simulink model "TosAeb"  (R2021a)
settings: Solver=FixedStepDiscrete  StartTime=0.0  StopTime=inf  FixedStep=0.01  RelTol=1e-3
undefined symbols (not defined in the file or in its folder): ACCModSts, AccSts1, AEB_ObjectMovingOrientation, AEB_ObjectMovingState, CN_BTCActive, CN_CurrentGearR, CN_CurrentGearR_DHT, CN_PTCActive, CN_VDCActive, CN_VSC_EspOff, CN_VSC_FCW_AEB_Error, CN_VSC_FCW_AEB_NoDispay, CN_VSC_FCW_AEB_PerfmDgdtn, CN_VSC_FCW_AEB_TempUnAvlbl, CN_VSC_VehStandstill, CNaccelAvoidMax, CNaccelAvoidMin, CNbrakePedalPress, CNcomfortStrLeftAccelMax, CNcomfortStrLeftAccelMin, CNcomfortStrRightAccelMax, CNcomfortStrRightAccelMin, CNDrvDoorOpen, CNDrvModeEco, CNDrvModeNorm, CNDrvModeOther, CNDrvModeSport, CNDrvSBRreminder, CNNull, CNobjInvalidID, CNPassDoorOpen, CNPassSBRreminder, CNPEBSAccelNegInf, CNPEBSAccelNull, CNPEBSTimeInf, CNPEBSTimeNull, CNSTOPMAX, CNSTOPNULL, CNsubjWidth, CNSwtOFF, CNSwtON, CNTTCMAX, CNTTCNULL, CNVehConfigAEBCycNone, CNVehConfigAEBNone, CNVehConfigAEBPedNone, CNVehConfigFCWNone, CNVehConfigJANone, CollisionSeverity, FALSE, FCWSnvty, JACycObjCalcInfoDefaultParams, JAPedObjCalcInfoDefaultParams, JAVehObjCalcInfoDefaultParams, latCycObjCalcInfoDefaultParams, latPedObjCalcInfoDefaultParams, longObjCalcInfoDefaultParams, ModSts, movingStatus, ObjectType ... (+1112)
libraries: simulink x3031, AEBSFunctionLib x50, Ta_Library x33, AEBSStateMachineLib x23, Mylibrary x12, simulink_extras x7, ObjSelect_lib x3, dspstat3 x2
(part 9 of 102)

== system TosAeb/FcwAebJa_Step_sys/ALG_AEBSystem/ALG_AEBController/AEB_StateMachineCalcApp/JA_AEBStateMachine ==
  note: Add New
  InputSignal : Inport
  JA_PTW_AEBfullStateMachine : Subsystem
  JA_PTW_PrefillStateMachine : Subsystem
  JA_cycAEBfullStateMachine : Subsystem
  JA_cycPrefillStateMachine : Subsystem
  JA_pedAEBfullStateMachine : Subsystem
  JA_pedPrefillStateMachine : Subsystem
  JA_vehAEBStateMachine : Subsystem
  JA_vehPrefillStateMachine : Subsystem
  Bus Element Out : Outport  PortName=JA_AEBRawModSts  Element=JA_vehPrefillRawModSts
  Bus Element Out1 : Outport  PortName=JA_AEBRawModSts  Element=JA_vehAEBRawModSts
  Bus Element Out2 : Outport  PortName=JA_AEBRawModSts  Element=JA_pedPrefillRawModSts
  Bus Element Out3 : Outport  PortName=JA_AEBRawModSts  Element=JA_pedAEBRawModSts
  Bus Element Out8 : Outport  PortName=JA_AEBRawModSts  Element=JA_cycPrefillRawModSts
  Bus Element Out9 : Outport  PortName=JA_AEBRawModSts  Element=JA_cycAEBRawModSts
  Bus Element Out12 : Outport  PortName=JA_AEBRawModSts  Element=JA_PTW_PrefillRawModSts
  Bus Element Out13 : Outport  PortName=JA_AEBRawModSts  Element=JA_PTW_AEBRawModSts
  Bus Element Out4 : Outport  Port=2  PortName=JA_AEBModSts  Element=JA_vehPrefillModSts
  Bus Element Out5 : Outport  Port=2  PortName=JA_AEBModSts  Element=JA_vehAEBModSts
  Bus Element Out6 : Outport  Port=2  PortName=JA_AEBModSts  Element=JA_pedPrefillModSts
  Bus Element Out7 : Outport  Port=2  PortName=JA_AEBModSts  Element=JA_pedAEBModSts
  Bus Element Out10 : Outport  Port=2  PortName=JA_AEBModSts  Element=JA_cycPrefillModSts
  Bus Element Out11 : Outport  Port=2  PortName=JA_AEBModSts  Element=JA_cycAEBModSts
  Bus Element Out14 : Outport  Port=2  PortName=JA_AEBModSts  Element=JA_PTW_PrefillModSts
  Bus Element Out15 : Outport  Port=2  PortName=JA_AEBModSts  Element=JA_PTW_AEBModSts
  wires:
  InputSignal/1 -> JA_vehAEBStateMachine/1(inputSignal), JA_pedPrefillStateMachine/1(inputSignal), JA_cycPrefillStateMachine/1(inputSignal), JA_cycAEBfullStateMachine/1(inputSignal), JA_PTW_AEBfullStateMachine/1(inputSignal), JA_PTW_PrefillStateMachine/1(inputSignal), JA_pedAEBfullStateMachine/1(inputSignal), JA_vehPrefillStateMachine/1(inputSignal)
  JA_vehPrefillStateMachine/2(JA_vehPrefillModSts) -> Bus Element Out4/1  "JA_vehPrefillModSts"
  JA_vehAEBStateMachine/2(JA_vehAEBModSts) -> Bus Element Out5/1  "JA_vehAEBModSts"
  JA_pedAEBfullStateMachine/2(JA_pedAEBModSts) -> Bus Element Out7/1  "JA_pedAEBModSts"
  JA_pedPrefillStateMachine/2(JA_pedPrefillModSts) -> Bus Element Out6/1  "JA_pedPrefillModSts"
  JA_vehPrefillStateMachine/1(JA_vehPrefillRawModSts) -> Bus Element Out/1
  JA_vehAEBStateMachine/1(JA_vehAEBRawModSts) -> Bus Element Out1/1
  JA_pedPrefillStateMachine/1(JA_pedPrefillRawModSts) -> Bus Element Out2/1
  JA_pedAEBfullStateMachine/1(JA_pedAEBRawModSts) -> Bus Element Out3/1
  JA_cycAEBfullStateMachine/1(JA_cycAEBRawModSts) -> Bus Element Out9/1
  JA_cycPrefillStateMachine/1(JA_cycPrefillRawModSts) -> Bus Element Out8/1
  JA_cycPrefillStateMachine/2(JA_cycPrefillModSts) -> Bus Element Out10/1
  JA_cycAEBfullStateMachine/2(JA_cycAEBModSts) -> Bus Element Out11/1
  JA_PTW_AEBfullStateMachine/2(JA_PTW_AEBModSts) -> Bus Element Out15/1
  JA_PTW_PrefillStateMachine/2(JA_PTW_PrefillModSts) -> Bus Element Out14/1
  JA_PTW_PrefillStateMachine/1(JA_PTW_PrefillRawModSts) -> Bus Element Out12/1
  JA_PTW_AEBfullStateMachine/1(JA_PTW_AEBRawModSts) -> Bus Element Out13/1

== system TosAeb/FcwAebJa_Step_sys/ALG_AEBSystem/ALG_AEBController/AEB_StateMachineCalcApp/JaFctStsArb ==
  JA_AEBModSts : Inport
  BrkArb : Subsystem
  BrkReadyArb : Subsystem
  Bus Selector : BusSelector  OutputSignals=JA_vehAEBModSts,JA_pedAEBModSts,JA_cycAEBModSts,JA_PTW_AEBModSts
  Bus Selector2 : BusSelector  OutputSignals=JA_vehPrefillModSts,JA_pedPrefillModSts,JA_cycPrefillModSts,JA_PTW_PrefillModSts
  Bus Element Out : Outport  PortName=JA_AEBModStsArb  Element=JA_vehAEBModStsArb
  Bus Element Out1 : Outport  PortName=JA_AEBModStsArb  Element=JA_PTW_AEBModStsArb
  Bus Element Out2 : Outport  PortName=JA_AEBModStsArb  Element=JA_cycAEBModStsArb
  Bus Element Out3 : Outport  PortName=JA_AEBModStsArb  Element=JA_pedAEBModStsArb
  Bus Element Out4 : Outport  PortName=JA_AEBModStsArb  Element=JA_vehPrefillModStsArb
  Bus Element Out5 : Outport  PortName=JA_AEBModStsArb  Element=JA_PTW_PrefillModStsArb
  Bus Element Out6 : Outport  PortName=JA_AEBModStsArb  Element=JA_cycPrefillModStsArb
  Bus Element Out7 : Outport  PortName=JA_AEBModStsArb  Element=JA_pedPrefillModStsArb
  Bus Element Out8 : Outport  Port=2  PortName=vehFctStsArbSuppAborCond  Element=brkSuppAborCondArb
  Bus Element Out9 : Outport  Port=2  PortName=vehFctStsArbSuppAborCond  Element=brkReadySuppAborCondArb
  wires:
  BrkArb/5(brkSuppAborCondArb) -> Bus Element Out8/1  "brkSuppAborCondArb"
  BrkArb/6(brkActvCond) -> BrkReadyArb/1(brkActvCond)
  JA_AEBModSts/1 -> Bus Selector/1, Bus Selector2/1
  Bus Selector/4 -> BrkArb/4(JA_PTW_AEBModSts)  "<JA_PTW_AEBModSts>"
  Bus Selector/3 -> BrkArb/3(JA_cycAEBModSts)  "<JA_cycAEBModSts>"
  Bus Selector/2 -> BrkArb/2(JA_pedAEBModSts)  "<JA_pedAEBModSts>"
  Bus Selector/1 -> BrkArb/1(JA_vehAEBModSts)  "<JA_vehAEBModSts>"
  Bus Selector2/1 -> BrkReadyArb/2(JA_vehPrefillModSts)  "<JA_vehPrefillModSts>"
  Bus Selector2/2 -> BrkReadyArb/3(JA_pedPrefillModSts)  "<JA_pedPrefillModSts>"
  Bus Selector2/3 -> BrkReadyArb/4(JA_cycPrefillModSts)  "<JA_cycPrefillModSts>"
  Bus Selector2/4 -> BrkReadyArb/5(JA_PTW_PrefillModSts)  "<JA_PTW_PrefillModSts>"
  BrkReadyArb/1(brkReadySuppAborCondArb) -> Bus Element Out9/1  "brkReadySuppAborCondArb"
  BrkArb/4(JA_pedAEBModStsArb) -> Bus Element Out3/1  "JA_pedAEBModStsArb"
  BrkArb/1(JA_vehAEBModStsArb) -> Bus Element Out/1  "JA_vehAEBModStsArb"
  BrkArb/2(JA_PTW_AEBModStsArb) -> Bus Element Out1/1  "JA_PTW_AEBModStsArb"
  BrkArb/3(JA_cycAEBModStsArb) -> Bus Element Out2/1  "JA_cycAEBModStsArb"
  BrkReadyArb/2(JA_vehPrefillModStsArb) -> Bus Element Out4/1  "JA_vehPrefillModStsArb"
  BrkReadyArb/3(JA_PTW_PrefillModStsArb) -> Bus Element Out5/1  "JA_PTW_PrefillModStsArb"
  BrkReadyArb/4(JA_cycPrefillModStsArb) -> Bus Element Out6/1  "JA_cycPrefillModStsArb"
  BrkReadyArb/5(JA_pedPrefillModStsArb) -> Bus Element Out7/1  "JA_pedPrefillModStsArb"

== system TosAeb/FcwAebJa_Step_sys/ALG_AEBSystem/ALG_AEBController/AEB_StateMachineCalcApp/LatAEBStateMachine ==
  InputSignal : Inport
  cycAEBfullStateMachine : Subsystem
  cycAEBpartStateMachine : Subsystem
  cycPrefillStateMachine : Subsystem
  pedABAStateMachine : Subsystem
  pedAEBfullStateMachine : Subsystem
  pedPreWarningStateMachine : Subsystem
  pedPrefillStateMachine : Subsystem
  Bus Element Out8 : Outport  PortName=latAEBRawModSts  Element=pedPrefillRawModSts
  Bus Element Out7 : Outport  PortName=latAEBRawModSts  Element=pedPreWarningRawModSts
  Bus Element Out6 : Outport  PortName=latAEBRawModSts  Element=pedABARawModSts
  Bus Element Out5 : Outport  PortName=latAEBRawModSts  Element=pedAEBfullRawModSts
  Bus Element Out4 : Outport  PortName=latAEBRawModSts  Element=cycPrefillRawModSts
  Bus Element Out3 : Outport  PortName=latAEBRawModSts  Element=cycAEBpartRawModSts
  Bus Element Out2 : Outport  PortName=latAEBRawModSts  Element=cycAEBfullRawModSts
  Bus Element Out : Outport  Port=2  PortName=latAEBModSts  Element=pedPrefillModSts
  Out Bus Element : Outport  Port=2  PortName=latAEBModSts  Element=pedPreWarningModSts
  Out Bus Element1 : Outport  Port=2  PortName=latAEBModSts  Element=pedABAModSts
  Out Bus Element2 : Outport  Port=2  PortName=latAEBModSts  Element=cycPrefillModSts
  Out Bus Element3 : Outport  Port=2  PortName=latAEBModSts  Element=pedAEBfullModSts
  Out Bus Element4 : Outport  Port=2  PortName=latAEBModSts  Element=cycAEBpartModSts
  Out Bus Element5 : Outport  Port=2  PortName=latAEBModSts  Element=cycAEBfullModSts
  Bus Element Out1 : Outport  Port=3  PortName=latActiveStateTime  Element=pedPrefillWorkTime
  Out Bus Element6 : Outport  Port=3  PortName=latActiveStateTime  Element=pedPreWarningWorkTime
  Out Bus Element7 : Outport  Port=3  PortName=latActiveStateTime  Element=pedABAWorkTime
  Out Bus Element8 : Outport  Port=3  PortName=latActiveStateTime  Element=pedAEBfullWorkTime
  Out Bus Element9 : Outport  Port=3  PortName=latActiveStateTime  Element=cycPrefillWorkTime
  Out Bus Element10 : Outport  Port=3  PortName=latActiveStateTime  Element=cycAEBpartWorkTime
  Out Bus Element11 : Outport  Port=3  PortName=latActiveStateTime  Element=cycAEBfullWorkTime
  wires:
  InputSignal/1 -> cycAEBfullStateMachine/1(inputSignal), cycAEBpartStateMachine/1(inputSignal), cycPrefillStateMachine/1(inputSignal), pedAEBfullStateMachine/1(inputSignal), pedABAStateMachine/1(inputSignal), pedPreWarningStateMachine/1(inputSignal), pedPrefillStateMachine/1(inputSignal)
  pedPrefillStateMachine/2(pedPrefillModSts) -> Bus Element Out/1  "pedPrefillModSts"
  pedPreWarningStateMachine/2(pedPreWarningModSts) -> Out Bus Element/1  "pedPreWarningModSts"
  pedABAStateMachine/2(pedABAModSts) -> Out Bus Element1/1  "pedABAModSts"
  cycPrefillStateMachine/2(cycPrefillModSts) -> Out Bus Element2/1  "cycPrefillModSts"
  pedAEBfullStateMachine/2(pedAEBfullModSts) -> Out Bus Element3/1  "pedAEBfullModSts"
  cycAEBpartStateMachine/2(cycAEBpartModSts) -> Out Bus Element4/1  "cycAEBpartModSts"
  cycAEBfullStateMachine/2(cycAEBfullModSts) -> Out Bus Element5/1  "cycAEBfullModSts"
  pedPrefillStateMachine/3(pedPrefillFuncActiveTime) -> Bus Element Out1/1  "pedPrefillFuncActiveTime"
  pedPreWarningStateMachine/3(pedPreWarningFuncActiveTime) -> Out Bus Element6/1
  pedABAStateMachine/3(pedABAFuncActiveTime) -> Out Bus Element7/1
  cycAEBpartStateMachine/3(cycAEBpartFuncActiveTime) -> Out Bus Element10/1
  cycAEBfullStateMachine/3(cycAEBfullFuncActiveTime) -> Out Bus Element11/1
  cycPrefillStateMachine/3(cycPrefillFuncActiveTime) -> Out Bus Element9/1
  pedAEBfullStateMachine/3(pedAEBfullFuncActiveTime) -> Out Bus Element8/1
  cycAEBfullStateMachine/1(cycAEBfullRawModSts) -> Bus Element Out2/1
  cycAEBpartStateMachine/1(cycAEBpartRawModSts) -> Bus Element Out3/1
  cycPrefillStateMachine/1(cycPrefillRawModSts) -> Bus Element Out4/1
  pedAEBfullStateMachine/1(pedAEBfullRawModSts) -> Bus Element Out5/1
  pedABAStateMachine/1(pedABARawModSts) -> Bus Element Out6/1
  pedPreWarningStateMachine/1(pedPreWarningRawModSts) -> Bus Element Out7/1
  pedPrefillStateMachine/1(pedPrefillRawModSts) -> Bus Element Out8/1

== system TosAeb/FcwAebJa_Step_sys/ALG_AEBSystem/ALG_AEBController/AEB_StateMachineCalcApp/LatFctStsArb ==
  latAEBModSts : Inport
  BrkArb : Subsystem
  BrkReadyArb : Subsystem
  Bus Creator1 : BusCreator  Inputs=7
  Bus Creator2 : BusCreator  Inputs=3
  WarningArb : Subsystem
  latAEBModStsArb : Outport
  latFctStsArbSuppAborCond : Outport  Port=2
  wires:
  [brkActvCond] -> WarningArb/2(brkActvCond)
  Bus Creator1/1 -> latAEBModStsArb/1  "latAEBModStsArb"
  Bus Creator2/1 -> latFctStsArbSuppAborCond/1  "latFctStsArbSuppAborCond"
  BrkArb/5(brkSuppAborCondArb) -> Bus Creator2/3  "brkSuppAborCondArb"
  [brkReadySuppAborCond] -> Bus Creator2/1  "brkReadySuppAborCond"
  WarningArb/2(warningSuppAborCond) -> [warningSuppAborCond]
  [warningSuppAborCond] -> Bus Creator2/2  "warningSuppAborCond"
  BrkReadyArb/4(brkReadySuppAborCond) -> [brkReadySuppAborCond]
  BrkArb/1(brkActvCond) -> BrkReadyArb/2(brkActvCond), [brkActvCond]
  latAEBModSts/1 -> WarningArb/1(vehAEBModSts), BrkArb/1(vehAEBModSts), BrkReadyArb/1(latAEBModSts)
  WarningArb/1(pedPreWarningModStsArb) -> Bus Creator1/1  "pedPreWarningModStsArb"
  BrkReadyArb/1(pedPrefillModStsArb) -> Bus Creator1/2  "pedPrefillModStsArb"
  BrkReadyArb/2(cycPrefillModStsArb) -> Bus Creator1/3  "cycPrefillModStsArb"
  BrkReadyArb/3(pedABAModStsArb) -> Bus Creator1/4  "pedABAModStsArb"
  BrkArb/4(pedAEBfullModStsArb) -> Bus Creator1/7  "pedAEBfullModStsArb"
  BrkArb/3(cycAEBfullModStsArb) -> Bus Creator1/6  "cycAEBfullModStsArb"
  BrkArb/2(cycAEBpartModStsArb) -> Bus Creator1/5  "cycAEBpartModStsArb"

== system TosAeb/FcwAebJa_Step_sys/ALG_AEBSystem/ALG_AEBController/AEB_StateMachineCalcApp/VehAEBStateMachine ==
  InputSignal : Inport
  ABAStateMachine : Subsystem
  AEBfullStateMachine : Subsystem
  AEBpartStateMachine : Subsystem
  ActuteWarningStateMachine : Subsystem
  EBAStateMachine : Subsystem
  LatentWarningStateMachine : Subsystem
  PreWarningStateMachine : Subsystem
  PrefillStateMachine : Subsystem
  Bus Element Out : Outport  PortName=vehRawModSts  Element=vehPrefillRawModSts
  Bus Element Out1 : Outport  PortName=vehRawModSts  Element=vehPreWarningRawModSts
  Bus Element Out2 : Outport  PortName=vehRawModSts  Element=vehAcuteWarningRawModSts
  Bus Element Out3 : Outport  PortName=vehRawModSts  Element=vehAEBpartRawModSts
  Bus Element Out4 : Outport  PortName=vehRawModSts  Element=vehAEBfullRawModSts
  Bus Element Out5 : Outport  PortName=vehRawModSts  Element=vehEBARawModSts
  Bus Element Out6 : Outport  PortName=vehRawModSts  Element=vehABARawModSts
  Bus Element Out7 : Outport  PortName=vehRawModSts  Element=vehLatentWarningRawModSts
  Bus Element Out8 : Outport  Port=2  PortName=vehAEBModSts  Element=vehPrefillModSts
  Bus Element Out9 : Outport  Port=2  PortName=vehAEBModSts  Element=vehPreWarningModSts
  Bus Element Out10 : Outport  Port=2  PortName=vehAEBModSts  Element=vehAcuteWarningModSts
  Bus Element Out11 : Outport  Port=2  PortName=vehAEBModSts  Element=vehAEBpartModSts
  Bus Element Out12 : Outport  Port=2  PortName=vehAEBModSts  Element=vehAEBfullModSts
  Bus Element Out13 : Outport  Port=2  PortName=vehAEBModSts  Element=vehEBAModSts
  Bus Element Out14 : Outport  Port=2  PortName=vehAEBModSts  Element=vehABAModSts
  Bus Element Out15 : Outport  Port=2  PortName=vehAEBModSts  Element=vehLatentWarningModSts
  wires:
  InputSignal/1 -> PreWarningStateMachine/1(inputSignal), ActuteWarningStateMachine/1(inputSignal), AEBpartStateMachine/1(inputSignal), AEBfullStateMachine/1(inputSignal), EBAStateMachine/1(inputSignal), LatentWarningStateMachine/1(inputSignal), ABAStateMachine/1(inputSignal), PrefillStateMachine/1(inputSignal)
  LatentWarningStateMachine/2(vehLatentWarningModSts) -> Bus Element Out15/1  "vehLatentWarningModSts"
  PrefillStateMachine/2(vehPrefillModSts) -> Bus Element Out8/1  "vehPrefillModSts"
  PreWarningStateMachine/2(vehPreWarningModSts) -> Bus Element Out9/1  "vehPreWarningModSts"
  ABAStateMachine/2(vehABAModSts) -> Bus Element Out14/1  "vehABAModSts"
  AEBfullStateMachine/2(vehAEBfullModSts) -> Bus Element Out12/1  "vehAEBfullModSts"
  AEBpartStateMachine/2(vehAEBpartModSts) -> Bus Element Out11/1  "vehAEBpartModSts"
  ActuteWarningStateMachine/2(vehAcuteWarningModSts) -> Bus Element Out10/1  "vehAcuteWarningModSts"
  EBAStateMachine/2(vehEBAModSts) -> Bus Element Out13/1  "vehEBAModSts"
  PrefillStateMachine/1(vehPrefillRawModSts) -> Bus Element Out/1
  PreWarningStateMachine/1(vehPreWarningRawModSts) -> Bus Element Out1/1
  ActuteWarningStateMachine/1(vehAcuteWarningRawModSts) -> Bus Element Out2/1
  AEBpartStateMachine/1(vehAEBpartRawModSts) -> Bus Element Out3/1
  AEBfullStateMachine/1(vehAEBfullRawModSts) -> Bus Element Out4/1
  EBAStateMachine/1(vehEBARawModSts) -> Bus Element Out5/1
  ABAStateMachine/1(vehABARawModSts) -> Bus Element Out6/1
  LatentWarningStateMachine/1(vehLatentWarningRawModSts) -> Bus Element Out7/1

== system TosAeb/FcwAebJa_Step_sys/ALG_AEBSystem/ALG_AEBController/AEB_StateMachineCalcApp/VehFCTModStsArb ==
  vehAEBModSts : Inport
  BrkArb : Subsystem
  BrkReadyArb : Subsystem
  Bus Creator1 : BusCreator  Inputs=8
  Bus Creator2 : BusCreator  Inputs=3
  WarningArb : Subsystem
  vehAEBModStsArb : Outport
  vehFctStsArbSuppAborCond : Outport  Port=2
  wires:
  vehAEBModSts/1 -> BrkArb/1(vehAEBModSts), BrkReadyArb/1(VehAEBModSts), WarningArb/1(vehAEBModSts)
  BrkArb/1(brkActvCond) -> [brkActvCond], BrkReadyArb/2(brkActvCond)
  BrkReadyArb/1(brkReadyActvCond) -> WarningArb/2(brkReadyActvCond)
  WarningArb/2(preWarningModStsArb) -> Bus Creator1/2  "preWarningModStsArb"
  BrkArb/4(EBAModStsArb) -> Bus Creator1/8  "EBAModStsArb"
  WarningArb/3(acuteWarningModStsArb) -> Bus Creator1/3  "acuteWarningModStsArb"
  WarningArb/1(latentWarningModStsArb) -> Bus Creator1/1  "latentWarningModStsArb"
  BrkArb/2(AEBpartModStsArb) -> Bus Creator1/6  "AEBpartModStsArb"
  BrkArb/3(AEBfullModStsArb) -> Bus Creator1/7  "AEBfullModStsArb"
  BrkReadyArb/4(brkReadySuppAborCond) -> [brkReadySuppAborCond]
  [warningSuppAborCond] -> Bus Creator2/2  "warningSuppAborCond"
  WarningArb/4(warningSuppAborCond) -> [warningSuppAborCond]
  [brkReadySuppAborCond] -> Bus Creator2/1  "brkReadySuppAborCond"
  BrkArb/5(brkSuppAborCondArb) -> Bus Creator2/3  "brkSuppAborCondArb"
  Bus Creator2/1 -> vehFctStsArbSuppAborCond/1  "FCTStsArbSuppAborCond"
  Bus Creator1/1 -> vehAEBModStsArb/1  "vehAEBModStsArb"
  [brkActvCond] -> WarningArb/3(brkActvCond)
  BrkReadyArb/2(prefillModStsArb) -> Bus Creator1/4  "prefillModStsArb"
  BrkReadyArb/3(ABAModStsArb) -> Bus Creator1/5  "ABAModStsArb"

== system TosAeb/FcwAebJa_Step_sys/ALG_AEBSystem/ALG_AEBController/AEB_VehicleStateCalcApp/CycSuppAborCondtionCalc ==
  InputSignal : Inport
  Bus Creator : BusCreator  Inputs=6  OutDataTypeStr=Bus: BusVSCCycFuncSuppAborCond
      settings: InheritFromInputs=off
  Bus Selector2 : BusSelector  OutputSignals=VSC_AEBFuncSwtSts.AEB_PedResp,VSC_FCW_AEB_FctSts.FCW_AEB_PedFctSts,vehMovingSts.vehUnstableSts,vehMovingSts.vehAssociatedSysStsSuppFlg,vehStateCalcSignalInput.vehConfigInfo.VehicleConfigAEBCyc,vehStateCalcSignalInput.blockTime.blockTimeBrakeReady
  Bus Selector5 : BusSelector  OutputSignals=VSC_AEBFuncSwtSts.AEB_PedResp,VSC_FCW_AEB_FctSts.FCW_AEB_PedFctSts,vehMovingSts.vehUnstableSts,vehMovingSts.vehAssociatedSysStsSuppFlg,vehStateCalcSignalInput.vehConfigInfo.VehicleConfigAEBCyc,vehStateCalcSignalInput.blockTime.blockTimeBrake
  Bus Selector6 : BusSelector  OutputSignals=VSC_AEBFuncSwtSts.AEB_PedResp,VSC_FCW_AEB_FctSts.FCW_AEB_PedFctSts,vehMovingSts.vehUnstableSts,vehMovingSts.vehAssociatedSysStsSuppFlg,vehStateCalcSignalInput.vehConfigInfo.VehicleConfigAEBCyc,vehStateCalcSignalInput.blockTime.blockTimeBrake
  CycAEBfullSuppAborConditonCalc : Subsystem
  CycAEBpartSuppAborConditonCalc : Subsystem
  CycPrefillSuppAborConditonCalc : Subsystem
  VSCCycFuncSuppAborCond : Outport  OutDataTypeStr=Bus: BusVSCCycFuncSuppAborCond
  wires:
  Bus Creator/1 -> VSCCycFuncSuppAborCond/1
  CycPrefillSuppAborConditonCalc/1(VSCCycPrefillSuppCond) -> Bus Creator/1  "VSCCycPrefillSuppCond"
  CycAEBfullSuppAborConditonCalc/2(VSCCycAEBfullAborCond) -> Bus Creator/6  "VSCCycAEBfullAborCond"
  CycPrefillSuppAborConditonCalc/2(VSCCycPrefillAborCond) -> Bus Creator/2  "VSCCycPrefillAborCond"
  CycAEBfullSuppAborConditonCalc/1(VSCCycAEBfullSuppCond) -> Bus Creator/5  "VSCCycAEBfullSuppCond"
  CycAEBpartSuppAborConditonCalc/1(VSCAEBpartSuppCond) -> Bus Creator/3  "VSCAEBpartSuppCond"
  CycAEBpartSuppAborConditonCalc/2(VSCAEBpartAborCond) -> Bus Creator/4  "VSCAEBpartAborCond"
  InputSignal/1 -> Bus Selector6/1, Bus Selector5/1, Bus Selector2/1
  Bus Selector2/1 -> CycPrefillSuppAborConditonCalc/1(AEB_PedResp)  "<AEB_PedResp>"
  Bus Selector2/2 -> CycPrefillSuppAborConditonCalc/2(FCW_AEB_PedFctSts)  "<FCW_AEB_PedFctSts>"
  Bus Selector2/3 -> CycPrefillSuppAborConditonCalc/3(vehUnstableStateFlg)  "<vehUnstableSts>"
  Bus Selector2/4 -> CycPrefillSuppAborConditonCalc/4(vehAssociatedSysStateSuppCond)  "<vehAssociatedSysStsSuppFlg>"
  Bus Selector2/5 -> CycPrefillSuppAborConditonCalc/5("vehConfigAEBCyc ")  "<VehicleConfigAEBCyc>"
  Bus Selector2/6 -> CycPrefillSuppAborConditonCalc/6(blockTimeBrakeReady)  "<blockTimeBrakeReady>"
  Bus Selector5/1 -> CycAEBpartSuppAborConditonCalc/1(VSCAEB_PedResp)  "<AEB_PedResp>"
  Bus Selector5/2 -> CycAEBpartSuppAborConditonCalc/2(FCW_AEB_PedFctSts)  "<FCW_AEB_PedFctSts>"
  Bus Selector5/3 -> CycAEBpartSuppAborConditonCalc/3(vehUnstableStateFlg)  "<vehUnstableSts>"
  Bus Selector5/4 -> CycAEBpartSuppAborConditonCalc/4(vehAssociatedSysStateSuppCond)  "<vehAssociatedSysStsSuppFlg>"
  Bus Selector5/5 -> CycAEBpartSuppAborConditonCalc/5("vehConfigAEBCyc ")  "<VehicleConfigAEBCyc>"
  Bus Selector5/6 -> CycAEBpartSuppAborConditonCalc/6(blockTimeBrake)  "<blockTimeBrake>"
  Bus Selector6/1 -> CycAEBfullSuppAborConditonCalc/1(VSCAEB_PedResp)  "<AEB_PedResp>"
  Bus Selector6/2 -> CycAEBfullSuppAborConditonCalc/2(FCW_AEB_PedFctSts)  "<FCW_AEB_PedFctSts>"
  Bus Selector6/3 -> CycAEBfullSuppAborConditonCalc/3(vehUnstableStateFlg)  "<vehUnstableSts>"
  Bus Selector6/4 -> CycAEBfullSuppAborConditonCalc/4(vehAssociatedSysStateSuppCond)  "<vehAssociatedSysStsSuppFlg>"
  Bus Selector6/5 -> CycAEBfullSuppAborConditonCalc/5("vehConfigAEBCyc ")  "<VehicleConfigAEBCyc>"
  Bus Selector6/6 -> CycAEBfullSuppAborConditonCalc/6(blockTimeBrakeReady)  "<blockTimeBrake>"

== system TosAeb/FcwAebJa_Step_sys/ALG_AEBSystem/ALG_AEBController/AEB_VehicleStateCalcApp/FuncErrorStateJudge ==
  note: 0x0:Trailer off
      | 0x1:Trailer on
  FCW_AEB_ErrorState : Inport
  AEB_Ped_ErrorState : Inport  Port=2
  AEB_JA_ErrorState : Inport  Port=3
  EspFuncOffSts : Inport  Port=4
  TrailerSts : Inport  Port=5
  Bus Creator : BusCreator  Inputs=5
  Bus Selector : BusSelector  OutputSignals=FCW_AEB_ErrorState,EspFuncOffSts,TrailerSts
  Bus Selector1 : BusSelector  OutputSignals=AEB_Ped_ErrorState,EspFuncOffSts,TrailerSts
  Bus Selector2 : BusSelector  OutputSignals=AEB_JA_ErrorState,EspFuncOffSts,TrailerSts
  FCW_AEB_JAFctStsCalc : Stateflow chart  TreatAsAtomicUnit=on
  FCW_AEB_PedFctStsCalc : Stateflow chart  TreatAsAtomicUnit=on
  FCW_AEB_VehFctStsCalc : Stateflow chart  TreatAsAtomicUnit=on
  FCW_AEB_VehFctSts : Outport
  FCW_AEB_PedFctSts : Outport  Port=2
  FCW_AEB_JAFctSts : Outport  Port=3
  wires:
  FCW_AEB_VehFctStsCalc/1(FCW_AEB_VehFctSts) -> FCW_AEB_VehFctSts/1
  FCW_AEB_JAFctStsCalc/1(FCW_AEB_JAFctSts) -> FCW_AEB_JAFctSts/1
  FCW_AEB_PedFctStsCalc/1(FCW_AEB_PedFctSts) -> FCW_AEB_PedFctSts/1
  AEB_Ped_ErrorState/1 -> Bus Creator/2  "AEB_Ped_ErrorState"
  FCW_AEB_ErrorState/1 -> Bus Creator/1  "FCW_AEB_ErrorState"
  AEB_JA_ErrorState/1 -> Bus Creator/3  "AEB_JA_ErrorState"
  EspFuncOffSts/1 -> Bus Creator/4  "EspFuncOffSts"
  TrailerSts/1 -> Bus Creator/5  "TrailerSts"
  Bus Creator/1 -> Bus Selector2/1, Bus Selector1/1, Bus Selector/1
  Bus Selector/1 -> FCW_AEB_VehFctStsCalc/1(FCW_AEB_ErrorState)  "<FCW_AEB_ErrorState>"
  Bus Selector/2 -> FCW_AEB_VehFctStsCalc/2(EspFuncOffSts)  "<EspFuncOffSts>"
  Bus Selector/3 -> FCW_AEB_VehFctStsCalc/3(TrailerSts)  "<TrailerSts>"
  Bus Selector1/1 -> FCW_AEB_PedFctStsCalc/1(FCW_AEB_ErrorState)  "<AEB_Ped_ErrorState>"
  Bus Selector1/2 -> FCW_AEB_PedFctStsCalc/2(EspFuncOffSts)  "<EspFuncOffSts>"
  Bus Selector1/3 -> FCW_AEB_PedFctStsCalc/3(TrailerSts)  "<TrailerSts>"
  Bus Selector2/1 -> FCW_AEB_JAFctStsCalc/1(FCW_AEB_ErrorState)  "<AEB_JA_ErrorState>"
  Bus Selector2/2 -> FCW_AEB_JAFctStsCalc/2(EspFuncOffSts)  "<EspFuncOffSts>"
  Bus Selector2/3 -> FCW_AEB_JAFctStsCalc/3(TrailerSts)  "<TrailerSts>"

== system TosAeb/FcwAebJa_Step_sys/ALG_AEBSystem/ALG_AEBController/AEB_VehicleStateCalcApp/FuncSwtStateJudge ==
  note: 0x00:Normal
      | 0x01:High sensitivity
      | 0x02:Low sensitivity
      | 0x03:Reserved
  AEBVehSwtReq : Inport
  AEBPedSwtReq : Inport  Port=2
  AEBJASwtReq : Inport  Port=3
  FCWSnvtySet : Inport  Port=4
  Compare To Constant : simulink/Logic and Bit Operations/Compare To Constant  relop===  const=CNSwtON  OutDataTypeStr=boolean
  Compare To Constant1 : simulink/Logic and Bit Operations/Compare To Constant  relop===  const=CNSwtON  OutDataTypeStr=boolean
  Compare To Constant2 : simulink/Logic and Bit Operations/Compare To Constant  relop===  const=CNSwtON  OutDataTypeStr=boolean
  Constant : Constant  Value=FCWSnvty.HMISettingMiddle
  Constant1 : Constant  Value=FCWSnvty.HMISettingLate
  Constant2 : Constant  Value=FCWSnvty.HMISettingEarly
  Constant3 : Constant  Value=P_AEB_AEBJaSwtReqConfig
  Constant4 : Constant  Value=CNSwtON
  Constant5 : Constant  Value=P_AEB_AEBPedSwtReqConfig
  Constant6 : Constant  Value=CNSwtON
  Constant7 : Constant  Value=CNSwtON
  Constant8 : Constant  Value=P_AEB_VehSwtReqConfig
  Multiport Switch : MultiPortSwitch  DataPortOrder=Specify indices  DataPortIndices={0,1,2}  DiagnosticForDefault=None
  Subsystem3 : Subsystem
  Switch : Switch  Criteria=u2 ~= 0
  Switch1 : Switch  Criteria=u2 ~= 0
  Switch2 : Switch  Criteria=u2 ~= 0
  AEB_VehResp : Outport
  AEB_PedResp : Outport  Port=2
  AEB_JAResp : Outport  Port=3
  FCWSnvty : Outport  Port=4
  FCWSnvtyALG : Outport  Port=5
  wires:
  Constant8/1 -> Switch2/2
  Constant7/1 -> Switch2/3
  Switch2/1 -> Compare To Constant/1
  AEBJASwtReq/1 -> Switch1/1
  Constant6/1 -> Switch1/3
  Constant3/1 -> Switch1/2
  Switch1/1 -> Compare To Constant2/1
  Switch/1 -> Compare To Constant1/1
  Constant4/1 -> Switch/3
  Constant5/1 -> Switch/2
  Constant1/1 -> Multiport Switch/4
  Constant2/1 -> Multiport Switch/3
  Constant/1 -> Multiport Switch/2
  Compare To Constant2/1 -> AEB_JAResp/1
  Compare To Constant1/1 -> AEB_PedResp/1
  Compare To Constant/1 -> AEB_VehResp/1
  AEBPedSwtReq/1 -> Switch/1
  AEBVehSwtReq/1 -> Switch2/1
  Multiport Switch/1 -> FCWSnvty/1
  Subsystem3/1(FCWLevelSts) -> Multiport Switch/1
  FCWSnvtySet/1 -> FCWSnvtyALG/1, Subsystem3/1(ALG_FCWLevelReq)

== system TosAeb/FcwAebJa_Step_sys/ALG_AEBSystem/ALG_AEBController/AEB_VehicleStateCalcApp/JaSuppAborCondtionCalc ==
  InputSignals : Inport
  Bus Creator : BusCreator  Inputs=12  OutDataTypeStr=Bus: BusVSC_JAFuncSuppAborCond
      settings: InheritFromInputs=off
  Bus Selector : BusSelector  OutputSignals=VSC_AEBFuncSwtSts.AEB_JAResp,VSC_FCW_AEB_FctSts.FCW_AEB_JAFctSts,vehMovingSts.vehUnstableSts,vehMovingSts.vehAssociatedSysStsSuppFlg,vehStateCalcSignalInput.vehConfigInfo.VehConfigAEBJA,vehStateCalcSignalInput.blockTime.blockTimeBrakeReady
  Bus Selector1 : BusSelector  OutputSignals=VSC_AEBFuncSwtSts.AEB_JAResp,VSC_FCW_AEB_FctSts.FCW_AEB_JAFctSts,vehMovingSts.vehUnstableSts,vehMovingSts.vehAssociatedSysStsSuppFlg,vehStateCalcSignalInput.vehConfigInfo.VehConfigAEBJA,vehStateCalcSignalInput.blockTime.blockTimeBrake
  Bus Selector2 : BusSelector  OutputSignals=VSC_AEBFuncSwtSts.AEB_PedResp,VSC_FCW_AEB_FctSts.FCW_AEB_JAFctSts,vehMovingSts.vehUnstableSts,vehMovingSts.vehAssociatedSysStsSuppFlg,vehStateCalcSignalInput.vehConfigInfo.VehicleConfigAEBPed,vehStateCalcSignalInput.blockTime.blockTimeBrakeReady
  Bus Selector3 : BusSelector  OutputSignals=VSC_AEBFuncSwtSts.AEB_PedResp,VSC_FCW_AEB_FctSts.FCW_AEB_JAFctSts,vehMovingSts.vehUnstableSts,vehMovingSts.vehAssociatedSysStsSuppFlg,vehStateCalcSignalInput.vehConfigInfo.VehicleConfigAEBPed,vehStateCalcSignalInput.blockTime.blockTimeBrake
  Bus Selector4 : BusSelector  OutputSignals=VSC_AEBFuncSwtSts.AEB_PedResp,VSC_FCW_AEB_FctSts.FCW_AEB_JAFctSts,vehMovingSts.vehUnstableSts,vehMovingSts.vehAssociatedSysStsSuppFlg,vehStateCalcSignalInput.vehConfigInfo.VehicleConfigAEBPed,vehStateCalcSignalInput.blockTime.blockTimeBrakeReady
  Bus Selector5 : BusSelector  OutputSignals=VSC_AEBFuncSwtSts.AEB_PedResp,VSC_FCW_AEB_FctSts.FCW_AEB_JAFctSts,vehMovingSts.vehUnstableSts,vehMovingSts.vehAssociatedSysStsSuppFlg,vehStateCalcSignalInput.vehConfigInfo.VehicleConfigAEBPed,vehStateCalcSignalInput.blockTime.blockTimeBrake
  JACycAEBSuppAborConditonCalc : Subsystem
  JACycPrefillSuppAborConditonCalc : Subsystem
  JAPedAEBSuppAborConditonCalc : Subsystem
  JAPedPrefillSuppAborConditonCalc : Subsystem
  JAVehAEBSuppAborConditonCalc : Subsystem
  JAVehPrefillSuppAborConditonCalc : Subsystem
  VSC_JAFuncSuppAborCond : Outport  OutDataTypeStr=Bus: BusVSC_JAFuncSuppAborCond
  wires:
  JACycAEBSuppAborConditonCalc/2(VSC_JACycAEBfullAborCond) -> Bus Creator/12  "VSC_JACycAEBfullAborCond"
  JACycAEBSuppAborConditonCalc/1(VSC_JACycAEBfullSuppCond) -> Bus Creator/11  "VSC_JACycAEBfullSuppCond"
  JACycPrefillSuppAborConditonCalc/2(VSC_JACycPrefillAborCond) -> Bus Creator/10  "VSC_JACycPrefillAborCond"
  JACycPrefillSuppAborConditonCalc/1(VSC_JACycPrefillSuppCond) -> Bus Creator/9  "VSC_JACycPrefillSuppCond"
  Bus Selector5/6 -> JACycAEBSuppAborConditonCalc/6(blockTimeBrake)  "<blockTimeBrake>"
  Bus Selector5/5 -> JACycAEBSuppAborConditonCalc/5("VehicleConfigAEBPed ")  "<VehicleConfigAEBPed>"
  Bus Selector5/4 -> JACycAEBSuppAborConditonCalc/4(vehAssociatedSysStateSuppCond)  "<vehAssociatedSysStsSuppFlg>"
  Bus Selector5/3 -> JACycAEBSuppAborConditonCalc/3(vehUnstableStateFlg)  "<vehUnstableSts>"
  Bus Selector5/2 -> JACycAEBSuppAborConditonCalc/2(FCW_AEB_JAFctSts)  "<FCW_AEB_JAFctSts>"
  Bus Selector5/1 -> JACycAEBSuppAborConditonCalc/1(AEB_PedResp)  "<AEB_PedResp>"
  Bus Selector4/6 -> JACycPrefillSuppAborConditonCalc/6(blockTimeBrakeReady)  "<blockTimeBrakeReady>"
  Bus Selector4/5 -> JACycPrefillSuppAborConditonCalc/5("VehicleConfigAEBPed ")  "<VehicleConfigAEBPed>"
  Bus Selector4/4 -> JACycPrefillSuppAborConditonCalc/4(vehAssociatedSysStateSuppCond)  "<vehAssociatedSysStsSuppFlg>"
  Bus Selector4/3 -> JACycPrefillSuppAborConditonCalc/3(vehUnstableStateFlg)  "<vehUnstableSts>"
  Bus Selector4/1 -> JACycPrefillSuppAborConditonCalc/1(AEB_PedResp)  "<AEB_PedResp>"
  Bus Selector4/2 -> JACycPrefillSuppAborConditonCalc/2(FCW_AEB_JAFctSts)  "<FCW_AEB_JAFctSts>"
  Bus Creator/1 -> VSC_JAFuncSuppAborCond/1
  JAPedAEBSuppAborConditonCalc/2(VSC_JAPedAEBfullAborCond) -> Bus Creator/8  "VSC_JAPedAEBfullAborCond"
  JAPedAEBSuppAborConditonCalc/1(VSC_JAPedAEBfullSuppCond) -> Bus Creator/7  "VSC_JAPedAEBfullSuppCond"
  JAVehPrefillSuppAborConditonCalc/1(VSC_JAVehPrefillSuppCond) -> Bus Creator/1  "VSC_JAVehPrefillSuppCond"
  JAPedPrefillSuppAborConditonCalc/2(VSC_JAPedPrefillAborCond) -> Bus Creator/6  "VSC_JAPedPrefillAborCond"
  JAVehPrefillSuppAborConditonCalc/2(VSC_JAVehPrefillAborCond) -> Bus Creator/2  "VSC_JAVehPrefillAborCond"
  JAPedPrefillSuppAborConditonCalc/1(VSC_JAPedPrefillSuppCond) -> Bus Creator/5  "VSC_JAPedPrefillSuppCond"
  JAVehAEBSuppAborConditonCalc/1(VSC_JAVehAEBfullSuppCond) -> Bus Creator/3  "VSC_JAVehAEBfullSuppCond"
  JAVehAEBSuppAborConditonCalc/2(VSC_JAVehAEBAborCond) -> Bus Creator/4  "VSC_JAVehAEBAborCond"
  Bus Selector2/2 -> JAPedPrefillSuppAborConditonCalc/2(FCW_AEB_JAFctSts)  "<FCW_AEB_JAFctSts>"
  Bus Selector2/1 -> JAPedPrefillSuppAborConditonCalc/1(AEB_PedResp)  "<AEB_PedResp>"
  Bus Selector2/3 -> JAPedPrefillSuppAborConditonCalc/3(vehUnstableStateFlg)  "<vehUnstableSts>"
  Bus Selector2/4 -> JAPedPrefillSuppAborConditonCalc/4(vehAssociatedSysStateSuppCond)  "<vehAssociatedSysStsSuppFlg>"
  Bus Selector2/5 -> JAPedPrefillSuppAborConditonCalc/5("VehicleConfigAEBPed ")  "<VehicleConfigAEBPed>"
  Bus Selector2/6 -> JAPedPrefillSuppAborConditonCalc/6(blockTimeBrakeReady)  "<blockTimeBrakeReady>"
  Bus Selector3/1 -> JAPedAEBSuppAborConditonCalc/1(AEB_PedResp)  "<AEB_PedResp>"
  Bus Selector3/2 -> JAPedAEBSuppAborConditonCalc/2(FCW_AEB_JAFctSts)  "<FCW_AEB_JAFctSts>"
  Bus Selector3/3 -> JAPedAEBSuppAborConditonCalc/3(vehUnstableStateFlg)  "<vehUnstableSts>"
  Bus Selector3/4 -> JAPedAEBSuppAborConditonCalc/4(vehAssociatedSysStateSuppCond)  "<vehAssociatedSysStsSuppFlg>"
  Bus Selector3/5 -> JAPedAEBSuppAborConditonCalc/5("VehicleConfigAEBPed ")  "<VehicleConfigAEBPed>"
  Bus Selector3/6 -> JAPedAEBSuppAborConditonCalc/6(blockTimeBrake)  "<blockTimeBrake>"
  Bus Selector1/6 -> JAVehAEBSuppAborConditonCalc/6(blockTimeBrake)  "<blockTimeBrake>"
  Bus Selector1/5 -> JAVehAEBSuppAborConditonCalc/5("vehConfigAEBJA ")  "<VehConfigAEBJA>"
  Bus Selector1/4 -> JAVehAEBSuppAborConditonCalc/4(vehAssociatedSysStateSuppCond)  "<vehAssociatedSysStsSuppFlg>"
  Bus Selector1/3 -> JAVehAEBSuppAborConditonCalc/3(vehUnstableStateFlg)  "<vehUnstableSts>"
  Bus Selector1/2 -> JAVehAEBSuppAborConditonCalc/2(FCW_AEB_JAFctSts)  "<FCW_AEB_JAFctSts>"
  Bus Selector1/1 -> JAVehAEBSuppAborConditonCalc/1(AEB_JAResp)  "<AEB_JAResp>"
  Bus Selector/6 -> JAVehPrefillSuppAborConditonCalc/6(blockTimeBrakeReady)  "<blockTimeBrakeReady>"
  Bus Selector/5 -> JAVehPrefillSuppAborConditonCalc/5("VehConfigAEBJA ")  "<VehConfigAEBJA>"
  Bus Selector/4 -> JAVehPrefillSuppAborConditonCalc/4(vehAssociatedSysStateSuppCond)  "<vehAssociatedSysStsSuppFlg>"
  Bus Selector/3 -> JAVehPrefillSuppAborConditonCalc/3(vehUnstableStateFlg)  "<vehUnstableSts>"
  Bus Selector/1 -> JAVehPrefillSuppAborConditonCalc/1(AEB_JAResp)  "<AEB_JAResp>"
  Bus Selector/2 -> JAVehPrefillSuppAborConditonCalc/2(FCW_AEB_JAFctSts)  "<FCW_AEB_JAFctSts>"
  InputSignals/1 -> Bus Selector/1, Bus Selector1/1, Bus Selector2/1, Bus Selector3/1, Bus Selector4/1, Bus Selector5/1

== system TosAeb/FcwAebJa_Step_sys/ALG_AEBSystem/ALG_AEBController/AEB_VehicleStateCalcApp/PedSuppAborCondtionCalc ==
  InputSignal : Inport
  Bus Creator : BusCreator  Inputs=8  OutDataTypeStr=Bus: BusVSCPedFuncSuppAborCond
      settings: InheritFromInputs=off
  Bus Selector : BusSelector  OutputSignals=VSC_AEBFuncSwtSts.AEB_PedResp,VSC_FCW_AEB_FctSts.FCW_AEB_PedFctSts,vehMovingSts.vehUnstableSts,vehMovingSts.vehAssociatedSysStsSuppFlg,vehStateCalcSignalInput.vehConfigInfo.VehicleConfigAEBPed,vehStateCalcSignalInput.blockTime.blockTimeBrakeReady
  Bus Selector1 : BusSelector  OutputSignals=VSC_AEBFuncSwtSts.AEB_PedResp,VSC_FCW_AEB_FctSts.FCW_AEB_PedFctSts,vehMovingSts.vehUnstableSts,vehMovingSts.vehAssociatedSysStsSuppFlg,vehStateCalcSignalInput.vehConfigInfo.VehicleConfigAEBPed,vehStateCalcSignalInput.blockTime.blockTimeBrakeReady
  Bus Selector3 : BusSelector  OutputSignals=VSC_AEBFuncSwtSts.AEB_PedResp,VSC_FCW_AEB_FctSts.FCW_AEB_PedFctSts,vehMovingSts.vehUnstableSts,vehMovingSts.vehAssociatedSysStsSuppFlg,vehStateCalcSignalInput.vehConfigInfo.VehicleConfigAEBPed,vehStateCalcSignalInput.blockTime.blockTimeWarning
  Bus Selector4 : BusSelector  OutputSignals=VSC_AEBFuncSwtSts.AEB_PedResp,VSC_FCW_AEB_FctSts.FCW_AEB_PedFctSts,vehMovingSts.vehUnstableSts,vehMovingSts.vehAssociatedSysStsSuppFlg,vehStateCalcSignalInput.vehConfigInfo.VehicleConfigAEBPed,vehStateCalcSignalInput.blockTime.blockTimeBrake
  PedABASuppAborConditonCalc : Subsystem
  PedAEBfullSuppAborConditonCalc : Subsystem
  PedPreWaringSuppAborConditonCalc : Subsystem
  PedPrefillSuppAborConditonCalc : Subsystem
  VSCPedFuncSuppAborCond : Outport  OutDataTypeStr=Bus: BusVSCPedFuncSuppAborCond
  wires:
  Bus Creator/1 -> VSCPedFuncSuppAborCond/1  "VSCPedFuncSuppAborCond"
  PedAEBfullSuppAborConditonCalc/2(VSCPedAEBfullAborCond) -> Bus Creator/8  "VSCPedAEBfullAborCond"
  PedAEBfullSuppAborConditonCalc/1(VSCPedAEBfullSuppCond) -> Bus Creator/7  "VSCPedAEBfullSuppCond"
  PedPrefillSuppAborConditonCalc/1(VSCPedPrefillSuppCond) -> Bus Creator/1  "VSCPedPrefillSuppCond"
  PedPrefillSuppAborConditonCalc/2(VSCPedPrefillAborCond) -> Bus Creator/2  "VSCPedPrefillAborCond"
  PedPreWaringSuppAborConditonCalc/2(VSCPedPreWaringAborCond) -> Bus Creator/6  "VSCPedPreWaringAborCond"
  PedPreWaringSuppAborConditonCalc/1(VSCPedPreWaringSuppCond) -> Bus Creator/5  "VSCPedPreWaringSuppCond"
  PedABASuppAborConditonCalc/1(VSCPedABASuppCond) -> Bus Creator/3  "VSCPedABASuppCond"
  PedABASuppAborConditonCalc/2(VSCPedABAAborCond) -> Bus Creator/4  "VSCPedABAAborCond"
  Bus Selector4/6 -> PedAEBfullSuppAborConditonCalc/6(blockTimeBrake)  "<blockTimeBrake>"
  Bus Selector4/5 -> PedAEBfullSuppAborConditonCalc/5("vehConfigAEBPed ")  "<VehicleConfigAEBPed>"
  Bus Selector4/4 -> PedAEBfullSuppAborConditonCalc/4(vehAssociatedSysStateSuppCond)  "<vehAssociatedSysStsSuppFlg>"
  Bus Selector4/3 -> PedAEBfullSuppAborConditonCalc/3(vehUnstableStateFlg)  "<vehUnstableSts>"
  Bus Selector4/2 -> PedAEBfullSuppAborConditonCalc/2(FCW_AEB_PedFctSts)  "<FCW_AEB_PedFctSts>"
  Bus Selector4/1 -> PedAEBfullSuppAborConditonCalc/1(AEB_PedResp)  "<AEB_PedResp>"
  Bus Selector3/6 -> PedPreWaringSuppAborConditonCalc/6(blockTimeWarning)  "<blockTimeWarning>"
  Bus Selector3/5 -> PedPreWaringSuppAborConditonCalc/5("vehConfigAEBPed ")  "<VehicleConfigAEBPed>"
  Bus Selector3/4 -> PedPreWaringSuppAborConditonCalc/4(vehAssociatedSysStateSuppCond)  "<vehAssociatedSysStsSuppFlg>"
  Bus Selector3/3 -> PedPreWaringSuppAborConditonCalc/3(vehUnstableStateFlg)  "<vehUnstableSts>"
  Bus Selector3/2 -> PedPreWaringSuppAborConditonCalc/2(FCW_AEB_PedFctSts)  "<FCW_AEB_PedFctSts>"
  Bus Selector3/1 -> PedPreWaringSuppAborConditonCalc/1(AEB_PedResp)  "<AEB_PedResp>"
  Bus Selector1/6 -> PedABASuppAborConditonCalc/6(blockTimeBrakeReady)  "<blockTimeBrakeReady>"
  Bus Selector1/5 -> PedABASuppAborConditonCalc/5("vehConfigAEBPed ")  "<VehicleConfigAEBPed>"
  Bus Selector1/4 -> PedABASuppAborConditonCalc/4(vehAssociatedSysStateSuppCond)  "<vehAssociatedSysStsSuppFlg>"
  Bus Selector1/3 -> PedABASuppAborConditonCalc/3(vehUnstableStateFlg)  "<vehUnstableSts>"
  Bus Selector1/2 -> PedABASuppAborConditonCalc/2(FCW_AEB_PedFctSts)  "<FCW_AEB_PedFctSts>"
  Bus Selector1/1 -> PedABASuppAborConditonCalc/1(AEB_PedResp)  "<AEB_PedResp>"
  Bus Selector/6 -> PedPrefillSuppAborConditonCalc/6(blockTimeBrakeReady)  "<blockTimeBrakeReady>"
  Bus Selector/5 -> PedPrefillSuppAborConditonCalc/5("vehConfigAEBPed ")  "<VehicleConfigAEBPed>"
  Bus Selector/4 -> PedPrefillSuppAborConditonCalc/4(vehAssociatedSysStateSuppCond)  "<vehAssociatedSysStsSuppFlg>"
  Bus Selector/1 -> PedPrefillSuppAborConditonCalc/1(AEB_PedResp)  "<AEB_PedResp>"
  Bus Selector/3 -> PedPrefillSuppAborConditonCalc/3(vehUnstableStateFlg)  "<vehUnstableSts>"
  Bus Selector/2 -> PedPrefillSuppAborConditonCalc/2(FCW_AEB_PedFctSts)  "<FCW_AEB_PedFctSts>"
  InputSignal/1 -> Bus Selector/1, Bus Selector1/1, Bus Selector3/1, Bus Selector4/1

== system TosAeb/FcwAebJa_Step_sys/ALG_AEBSystem/ALG_AEBController/AEB_VehicleStateCalcApp/VehMovingStateJudge ==
  VDCActive : Inport
  BTCActive : Inport  Port=2
  PTCActive : Inport  Port=3
  CURRENT_GEAR : Inport  Port=4
  HCU_GearSts : Inport  Port=5
  EspFuncOffSts : Inport  Port=6
  DrivingModeDis : Inport  Port=7
  DriverDoorSts : Inport  Port=8
  PassengerDoorSts : Inport  Port=9
  DriverSBR : Inport  Port=10
  PassSBR : Inport  Port=11
  EngState : Inport  Port=12
  HCU_PowertrainSts : Inport  Port=13
  HCU_Start_StopSts : Inport  Port=14
  subjVel : Inport  Port=15
  TrailerSts : Inport  Port=16
  Compare To Constant1 : simulink/Logic and Bit Operations/Compare To Constant  relop===  const=CN_VSC_EspOff  OutDataTypeStr=boolean
  Compare To Constant10 : simulink/Logic and Bit Operations/Compare To Constant  relop===  const=CN_VDCActive  OutDataTypeStr=boolean
  Compare To Constant11 : simulink/Logic and Bit Operations/Compare To Constant  relop===  const=CN_BTCActive  OutDataTypeStr=boolean
  Compare To Constant12 : simulink/Logic and Bit Operations/Compare To Constant  relop===  const=CN_PTCActive  OutDataTypeStr=boolean
  Compare To Constant2 : simulink/Logic and Bit Operations/Compare To Constant  relop===  const=CNDrvModeEco  OutDataTypeStr=boolean
  Compare To Constant3 : simulink/Logic and Bit Operations/Compare To Constant  relop===  const=CNDrvModeNorm  OutDataTypeStr=boolean
  Compare To Constant4 : simulink/Logic and Bit Operations/Compare To Constant  relop===  const=CNDrvModeSport  OutDataTypeStr=boolean
  Compare To Constant5 : simulink/Logic and Bit Operations/Compare To Constant  relop===  const=CNDrvDoorOpen  OutDataTypeStr=boolean
  Compare To Constant6 : simulink/Logic and Bit Operations/Compare To Constant  relop===  const=CNPassSBRreminder  OutDataTypeStr=boolean
  Compare To Constant7 : simulink/Logic and Bit Operations/Compare To Constant  relop===  const=CNDrvSBRreminder  OutDataTypeStr=boolean
  Compare To Constant8 : simulink/Logic and Bit Operations/Compare To Constant  relop===  const=CNPassDoorOpen  OutDataTypeStr=boolean
  Compare To Constant9 : simulink/Logic and Bit Operations/Compare To Constant  relop===  const=TRUE  OutDataTypeStr=boolean
  Constant : Constant  Value=FALSE
  Constant1 : Constant  Value=TRUE
  EngStateSuppFlg_HCU1 : Subsystem  VariantControl=P_AEB_PowerTrainConfig == uint8(1)
  Logical Operator : Logic  Operator=OR  Inputs=3  AllPortsSameDT=off  OutDataTypeStr=boolean
  Logical Operator2 : Logic  Operator=OR  Inputs=3  AllPortsSameDT=off  OutDataTypeStr=boolean
  Logical Operator3 : Logic  Operator=OR  Inputs=4  AllPortsSameDT=off  OutDataTypeStr=boolean
  Logical Operator4 : Logic  Operator=OR  Inputs=6  AllPortsSameDT=off  OutDataTypeStr=boolean
  Subsystem1 : Subsystem  VariantControl=P_AEB_PowerTrainConfig == 1
  Switch : Switch  Criteria=u2 ~= 0
  vehUnstableStateFlg : Outport
  vehAssociatedSysStsSuppFlg : Outport  Port=2
  wires:
  HCU_GearSts/1 -> Subsystem1/2(HCU_GearSts)
  CURRENT_GEAR/1 -> Subsystem1/1(CURRENT_GEAR)
  Compare To Constant9/1 -> Logical Operator4/6  "TrailerStsSuppFlg0"
  TrailerSts/1 -> Compare To Constant9/1
  subjVel/1 -> EngStateSuppFlg_HCU1/4(subjVel)
  HCU_Start_StopSts/1 -> EngStateSuppFlg_HCU1/3(HCU_Start_StopSts)
  HCU_PowertrainSts/1 -> EngStateSuppFlg_HCU1/2(HCU_PowertrainSts)
  Constant1/1 -> Switch/3
  Constant/1 -> Switch/1
  Logical Operator2/1 -> Switch/2
  Compare To Constant6/1 -> Logical Operator3/4
  Compare To Constant7/1 -> Logical Operator3/3
  Compare To Constant8/1 -> Logical Operator3/2
  Compare To Constant12/1 -> Logical Operator/3
  Compare To Constant11/1 -> Logical Operator/2
  Compare To Constant10/1 -> Logical Operator/1
  Logical Operator4/1 -> vehAssociatedSysStsSuppFlg/1  "vehAssociatedSysStsSuppFlg"
  EngStateSuppFlg_HCU1/1(EngStateSuppFlg) -> Logical Operator4/5  "EngStateSuppFlg0"
  Subsystem1/1(TCU_OperatingStateSuppFlg) -> Logical Operator4/1  "TCUOperatingStateSuppFlg0"
  Compare To Constant1/1 -> Logical Operator4/2  "ESPOperatingStateSuppFlg0"
  Logical Operator3/1 -> Logical Operator4/4  "bodyCtrlSuppFlg0"
  Switch/1 -> Logical Operator4/3  "DrvModOperatingStateSuppFlg0"
  EngState/1 -> EngStateSuppFlg_HCU1/1(EngState)
  Compare To Constant5/1 -> Logical Operator3/1
  PassengerDoorSts/1 -> Compare To Constant8/1
  DriverSBR/1 -> Compare To Constant7/1
  PassSBR/1 -> Compare To Constant6/1
  DriverDoorSts/1 -> Compare To Constant5/1
  Compare To Constant2/1 -> Logical Operator2/1
  Compare To Constant4/1 -> Logical Operator2/3
  Compare To Constant3/1 -> Logical Operator2/2
  DrivingModeDis/1 -> Compare To Constant3/1, Compare To Constant4/1, Compare To Constant2/1
  EspFuncOffSts/1 -> Compare To Constant1/1
  Logical Operator/1 -> vehUnstableStateFlg/1  "vehUnstableStateFlg"
  PTCActive/1 -> Compare To Constant12/1
  VDCActive/1 -> Compare To Constant10/1
  BTCActive/1 -> Compare To Constant11/1
